simulink library "Renault_Library"  (Simulink 8.2)
settings: Solver=ode3  StartTime=0.0  StopTime=10.0  FixedStep=0.020  RelTol=1e-3
undefined symbols (not defined in the file or in its folder): ABC, AssystemProposal, b, chkval, CoeffA_C, CoeffB_C, CoeffMean_C, ConfTime_C, DiagDelay_C, DividePercent, FailureConfActive_C, FallingEdgeDuration_C, HighLevelThresholdVoltage_C, HighLevelVoltage_C, HighLevelVoltageTunable_C, InitValue_C, InputVoltRangeMax_C, InputVoltRangeMin_C, LowActiveContact_C, LowLevelThresholdVoltage_C, LowLevelVoltage_C, MaxOperatingVoltage_C, MinOperatingVoltage_C, mV2V_conversion, OUT1, OutOfRangeHigh_C, OutOfRangeLow_C, PinSupplySaturationMax, PinSupplySaturationMin, Res1Value_C, Res2Value_C, Res3Value_C, RisingEdgeDuration_C, RSA_CANSignalFailMonitoring, SampleTime_C, service14Table, service19Table, service22Table, service24Table, service28Table, service2ETable, service2FTable, service31Table, service87Table, SizeXStateDID2FTable, SizeXTable14, SizeXTable19, SizeXTable22, SizeXTable24, SizeXTable28, SizeXTable2E, SizeXTable2F, SizeXTable31, SizeXTable87, SizeYStateDID2FTable, SizeYTable14, SizeYTable19, SizeYTable22, SizeYTable24, SizeYTable28 ... (+22)
undefined symbols (not defined in the file): BatteryVoltage_ActivationThreshold_C, FrameStatus_AbsentValue_C, FrameStatus_ConfAbsentValue_C, MemThreshold_C, NAMEOFTHEMODEL_SAMPLE_TIME_CST, NbAbs1_C, NbConfAbs1_C, NbConfPres1_C, NbPres1_C, NOMDUSIGNAL_FaultConfTime_C, NOMDUSIGNAL_FaultRehabTime_C, NOMDUSIGNAL_switchvalue_C, NOMDUSIGNAL_unavailablevalue_C, PeriodFrame1_C, ResetTime_C, WakeUpTime_C
libraries: Renault_Library x112, simulink x75
(part 2 of 32)

== system Renault_Library/Complex blocks/Logic & Bit Operations ==
  note: Logic & Bit Operations
  note: /!\         DO NOT USE THIS RED BLOCKS ANYMORE          /!\
      |
      |
      |
      |
      |
      |
      |
      |
      |
      |
      |
      |
      |
      |
      |
      |
      |
      |
      |
      |
      |
      |
      |
      |
      | .
  RSA_CompareToConstant_V01_04 : Subsystem  Tag=[LIB]  mask(RSA_CompareToConstant){relop===, Const=1, LogicOutDataTypeMode=boolean, ZeroCross=off}
      mask prompts: relop "Operator:"; Const "Constant value:"; LogicOutDataTypeMode "Output data type mode:"; ZeroCross "Enable zero-crossing detection"
      mask help:
      | Output will be one when the signal less than or equal to a constant otherwise it will be zero.
      |
      | The “Operator” parameter can have the following values:
      |       1. "==" — Determine whether the input is equal to Constant.
      |       2. "~=" — Determine whether the input is not equal to Constant.
      |       3. "<"  — Determine whether the input is less than Constant.
      |       4. "<=" — Determine whether the input is less than or equal to Constant.
      |       5. ">"  — Determine whether the input is greater than Constant.
      |       6. ">=" — Determine whether the input is greater than or equal to Constant.
      |
      | Note:
      |       1. Input Datatype of the block must NOT be "Double" and "Single" when "==" or "~="  is used
      |
      |
      | Version : 01.04
      mask init:
      | blkName = gcb;
      | 
      | if strcmp(get_param(gcbh,'LogicOutDataTypeMode'),'boolean')
      | 	setValue = 'boolean';
      | else
      | 	setValue = '''uint8''';
      | end
      | 
      | set_param([blkName,'/Compare'],'relop',relop, ...
      | 	'OutDataTypeStr', setValue, 'ZeroCross', ZeroCross);
      | 
      | str = [relop,' C']; %default
      | 
      | const_str = get_param(blkName, 'Const');
      | 
      | if isempty(strfind(const_str, ' ')) && isempty(strfind(const_str, ','))
      |     % scalar or single expression
      |     if isempty(str2num(const_str))
      |         %expression
      |         str = [relop, ' ', const_str];
      |     elseif length(Const) == 1
      |         str = [relop,' ',num2str(double(Const))];
      |     end
      | end
      settings: AttributesFormatString=%<MaskType> : Ver : 01.04\nOperator: = %<relop>\nConstant value: = %<Const>\nOutput data type mode: = %<LogicOutDataTypeMode>\nEnable zero-crossing detection: = %<ZeroCross>
      mask Help: eval(['open([','''\\F-khepri\shared\DIM-DCMAP-CGMP\66161\Shared_MatLab\Tools_DCMAP\Common_Library_MBDR\Library\Common_Library\Help\RLV_RSA_CompareToConstant_Spec_V1.04.pdf''','])'])
      mask TypeOptions relop: == | ~= | < | <= | >= | >
      mask TypeOptions LogicOutDataTypeMode: uint8 | boolean
      mask Callback ZeroCross: sl('zeroCrossEnable', gcbh);
      mask Display: disp(str)
  RSA_CompareToZero : Subsystem  Tag=[LIB]  mask(RSA_CompareToZero){relop===, LogicOutDataTypeMode=boolean, ZeroCross=on}
      mask prompts: relop "Operator:"; LogicOutDataTypeMode "Output data type mode:"; ZeroCross "Enable zero-crossing detection"
      mask help:
      | RSA_CompareToZero block compares an input signal to zero.
      | Specify how the input is compared to zero with the “Operator” parameter.
      | The “Operator” parameter can have the following values:
      |       1. "==" — Determine whether the input is equal to zero.
      |       2. "~=" — Determine whether the input is not equal to zero.
      |       3. "<"  — Determine whether the input is less than zero.
      |       4. "<=" — Determine whether the input is less than or equal to zero.
      |       5. ">"  — Determine whether the input is greater than zero.
      |       6. ">=" — Determine whether the input is greater than or equal to zero.
      |
      | The output is 0 if the comparison is false, and 1 if it is true.
      |
      | Version : 01.03
      mask init:
      | cons_zero = 0;
      | blkName = gcb;
      | 
      | if strcmp(get_param(gcbh,'LogicOutDataTypeMode'),'boolean')
      | 	setValue = 'boolean';
      | else
      |     setValue = '''uint8''';
      | end
      | 
      | set_param([blkName,'/Compare'],'relop',relop, ...
      | 	'OutDataTypeStr', setValue, 'ZeroCross', ZeroCross);
      settings: AttributesFormatString=%<MaskType> : Ver : 01.03\nOperator: %<relop>\nOutput data type mode: %<LogicOutDataTypeMode>\nEnable zero-crossing detection %<ZeroCross>
      mask Help: eval(['open([','''\\F-khepri\shared\DIM-DCMAP-CGMP\66161\Shared_MatLab\Tools_DCMAP\Common_Library_MBDR\Library\Common_Library\Help\RLV_RSA_CompareToZero_Spec_V1.03.pdf''','])'])
      mask TypeOptions relop: == | ~= | < | <= | >= | >
      mask TypeOptions LogicOutDataTypeMode: uint8 | boolean
      mask Display: disp([relop,' 0'])
  RSA_CompareToZero1 : Subsystem  Tag=[LIB]  mask(RSA_CompareToZero){relop===, LogicOutDataTypeMode=boolean, ZeroCross=on}
      mask prompts: relop "Operator:"; LogicOutDataTypeMode "Output data type mode:"; ZeroCross "Enable zero-crossing detection"
      mask help:
      | RSA_CompareToZero block compares an input signal to zero.
      | Specify how the input is compared to zero with the “Operator” parameter.
      | The “Operator” parameter can have the following values:
      |       1. "==" — Determine whether the input is equal to zero.
      |       2. "~=" — Determine whether the input is not equal to zero.
      |       3. "<"  — Determine whether the input is less than zero.
      |       4. "<=" — Determine whether the input is less than or equal to zero.
      |       5. ">"  — Determine whether the input is greater than zero.
      |       6. ">=" — Determine whether the input is greater than or equal to zero.
      |
      | The output is 0 if the comparison is false, and 1 if it is true.
      |
      | Note:
      |       1. Input Datatype of the block must NOT be "Double" and "Single" when "==" or "~="  is used
      |
      |
      | Version : 01.04
      mask init: same as Renault_Library/Complex blocks/Logic & Bit Operations/RSA_CompareToZero
      settings: AttributesFormatString=%<MaskType> : Ver : 01.04\nOperator: %<relop>\nOutput data type mode: %<LogicOutDataTypeMode>\nEnable zero-crossing detection %<ZeroCross>
      mask Help: eval(['open([','''\\F-khepri\shared\DIM-DCMAP-CGMP\66161\Shared_MatLab\Tools_DCMAP\Common_Library_MBDR\Library\Common_Library\Help\RLV_RSA_CompareToZero_Spec_V1.04.pdf''','])'])
      mask TypeOptions relop: == | ~= | < | <= | >= | >
      mask TypeOptions LogicOutDataTypeMode: uint8 | boolean
      mask Display: disp([relop,' 0'])
  RSA_DetectBooleanChange_v01_0 : Subsystem  Tag=[LIB]  mask(RSA_DetectBooleanChange){chkini=on, vinit=false}
      mask prompts: chkini "Enable first step detection"; vinit "Initial Value :"
      mask help:
      | Detect change in Boolean signal value.
      |
      | If the input does not equal its previous value, then output TRUE, otherwise output FALSE.
      |
      | To inhibit change detection at first simulation step, uncheck "Enable first step detection".
      |
      | Version: 01.0
      mask init:
      | if strcmp(get_param(gcb,'vinit'), 'false')
      |      set_param([gcb '/Delay_Input1'],'InitialCondition','false');
      | else
      |      set_param([gcb '/Delay_Input1'],'InitialCondition','true');
      | end
      settings: AttributesFormatString=|
      | %<MaskType> : Ver : 01.0
      | First step detection = %<chkini>
      | Initial Value : = %<vinit>
      mask Help: eval(['open([','''\\F-khepri\shared\DIM-DCMAP-CGMP\66161\Shared_MatLab\Tools_DCMAP\Common_Library_MBDR\Library\Common_Library\Help\RLV_RSA_DetectBooleanChange_V1_00.pdf''','])'])
      mask Callback chkini:
      | if strcmp(get_param(gcb,'chkini'),'on')
      |         set_param(gcb,'Maskvisibilities',{'on','on'});
      |         set_param(gcb,'attributesFormatString',sprintf('%%<MaskType> : Ver : 01.0\nFirst step detection = %%<chkini>\nInitial Value : = %%<vinit>'));
      | else
      |         set_param(gcb,'Maskvisibilities',{'on','off'});
      |         set_param(gcb,'attributesFormatString',sprintf('%%<MaskType> : Ver : 01.0\nFirst step detection = %%<chkini>'));
      | end
      mask TypeOptions vinit: false | true
      mask Callback vinit:
      | if strcmp(get_param(gcb,'vinit'), 'false')
      |      set_param([gcb '/Delay_Input1'],'InitialCondition','false');
      | else
      |      set_param([gcb '/Delay_Input1'],'InitialCondition','true');
      | end
      mask Display: fprintf(['U ~= U/z' '\nBoolean inputs'])
  RSA_DetectChange : Subsystem  Tag=[LIB]  mask(RSA_DetectChange){vinit=2}
      mask prompts: vinit "Initial condition:"
      mask help:
      | If the input does not equal its previous value, then output TRUE, otherwise output FALSE.  The initial condition determines the initial value of the previous input U/z.
      |
      | Version : 01.02
      settings: AttributesFormatString=%<MaskType> : Ver : 01.02\nInitial condition: %<vinit>
      mask Help: eval(['open([','''\\F-khepri\shared\DIM-DCMAP-CGMP\66161\Shared_MatLab\Tools_DCMAP\Common_Library_MBDR\Library\Common_Library\Help\RLV_RSA_DetectChange_Spec_V1.02.pdf''','])'])
      mask Display: fprintf('U ~= U/z')
  RSA_DetectChange_v01_03 : Subsystem  Tag=[LIB]  mask(RSA_DetectChange){}
      mask help:
      | If the input does not equal its previous value, then output TRUE, otherwise output FALSE.
      |
      | Version : 01.03
      mask init:
      | vinit = 0;
      settings: AttributesFormatString=%<MaskType> : Ver : 01.03
      mask Help: eval(['open([','''\\F-khepri\shared\DIM-DCMAP-CGMP\66161\Shared_MatLab\Tools_DCMAP\Common_Library_MBDR\Library\Common_Library\Help\RLV_RSA_DetectChange_Spec_V1.03.pdf''','])'])
      mask Display: fprintf('U ~= U/z')
  RSA_DetectChange_v01_04 : Subsystem  Tag=[LIB]  mask(RSA_DetectChange){chkini=on, vinit=0}
      mask prompts: chkini "Compare the Input with Initial Value"; vinit "Initial Value :"
      mask help:
      | If the input does not equal its previous value, then output TRUE, otherwise output FALSE.
      |
      | Version : 01.04
      settings: AttributesFormatString=%<MaskType> : Ver : 01.04\nCompare the Input with Initial Value = %<chkini>\nInitial Value : = %<vinit>
      mask Help: eval(['open([','''\\F-khepri\shared\DIM-DCMAP-CGMP\66161\Shared_MatLab\Tools_DCMAP\Common_Library_MBDR\Library\Common_Library\Help\RLV_RSA_DetectChange_Spec_V1.04.pdf''','])'])
      mask Callback chkini:
      | chkval = false;
      | if strcmp(get_param(gcb,'chkini'),'on')
      |     set_param(gcb,'MaskEnables',{'on','on'});
      |     chkval = true;
      | else
      |     set_param(gcb,'MaskEnables',{'on','off'});
      | end
      mask Display: fprintf('U ~= U/z')
  RSA_DetectChange_v01_05 : Subsystem  Tag=[LIB]  mask(RSA_DetectChange){chkini=on, vinit=0, tolerance=Absolute Zero, user_tol=0}
      mask prompts: chkini "Compare the Input with Initial Value"; vinit "Initial Value :"; tolerance "Tolerance for Input"; user_tol "User Defined Tolerance"
      mask help:
      | If the input does not equal its previous value, then output TRUE, otherwise output FALSE.
      | Initial condition of the output is FALSE.
      |
      | NOTE: The check for the initial condition can be inhibited by unchecking the checkbox "Compare the input with Initial Value"
      |
      | Version : 01.05
      mask init:
      | if(strcmp(get_param(gcb,'chkini'),'on'))
      |      set_param([gcb '/From'],'GotoTag','fromRelOpe');
      | else
      |      set_param([gcb '/From'],'GotoTag','vchk_val');
      | end
      | 
      | switch tolerance
      |    case 1
      |        tol=eps('double');
      |    case 2
      |        tol=eps('single');
      |    case 3
      |        tol=0;
      |     case 4
      |        tol = user_tol;
      | end
      settings: AttributesFormatString=|
      | %<MaskType> : Ver : 01.05
      | Compare the Input with Initial Value = %<chkini>
      | Initial Value : = %<vinit>
      | Tolerance : %<tolerance>
      mask Help: eval(['open([','''\\F-khepri\shared\DIM-DCMAP-CGMP\66161\Shared_MatLab\Tools_DCMAP\Common_Library_MBDR\Library\Common_Library\Help\RLV_RSA_DetectChange_Spec_V1.05.pdf''','])'])
      mask Callback chkini:
      | if strcmp(get_param(gcb,'chkini'),'on')
      |     if (strcmp(get_param(gcb,'tolerance'),'User Defined'))
      |         set_param(gcb,'Maskvisibilities',{'on','on','on','on'});
      |         set_param(gcb,'attributesFormatString',sprintf('%%<MaskType> : Ver : 01.05\nCompare the Input with Initial Value = %%<chkini>\nInitial Value : = %%<vinit>\nTolerance : %%<tolerance>\nUser Defined Tol : %%<user_tol>'));
      |     else
      |         set_param(gcb,'Maskvisibilities',{'on','on','on','off'});
      |         set_param(gcb,'attributesFormatString',sprintf('%%<MaskType> : Ver : 01.05\nCompare the Input with Initial Value = %%<chkini>\nInitial Value : = %%<vinit>\nTolerance : %%<tolerance>\n'));
      |     end
      | else
      |     if (strcmp(get_param(gcb,'tolerance'),'User Defined'))
      |         set_param(gcb,'Maskvisibilities',{'on','off','on','on'});
      |         set_param(gcb,'attributesFormatString',sprintf('%%<MaskType> : Ver : 01.05\nCompare the Input with Initial Value = %%<chkini>\nTolerance : %%<tolerance>\nUser Defined Tol : %%<user_tol>'));
      |     else
      |         set_param(gcb,'Maskvisibilities',{'on','off','on','off'});
      |         set_param(gcb,'attributesFormatString',sprintf('%%<MaskType> : Ver : 01.05\nCompare the Input with Initial Value = %%<chkini>\nTolerance : %%<tolerance>\n'));
      |     end
      |     vinit=0;
      | end
      mask TypeOptions tolerance: double tolerance - eps('double') | single tolerance - eps('single') | Absolute Zero | User Defined
      mask Callback tolerance:
      | % if strcmp(get_param(gcb,'chkini'),'on')
      | % set_param(gcb,'AttributesFormatString',sprintf('%%<MaskType> : Ver : 01.05\nCompare the Input with Initial Value = %%<chkini>\nInitial Value : = %%<vinit> \nTolerance : %%<tolerance>'));
      | % else
      | % set_param(gcb,'AttributesFormatString',sprintf('%%<MaskType> : Ver : 01.05\nCompare the Input with Initial Value = %%<chkini> \nTolerance : %%<tolerance>'));
      | % end
      | % switch get_param(gcb,'tolerance')
      | %   case 'double tolerance - eps(''double'')'
      | %       set_param(gcb,'MaskVisibilities',{'on',get_param(gcb,'chkini'), 'on','off'});
      | %   case 'single tolerance - eps(''single'')'
      | %       set_param(gcb,'MaskVisibilities',{'on',get_param(gcb,'chkini'), 'on','off'});
      | %   case 'Absolute Zero'
      | %       set_param(gcb,'MaskVisibilities',{'on',get_param(gcb,'chkini'), 'on','off'});
      | %    case'User Defined'
      | %        if strcmp(get_param(gcb,'chkini'),'on')
      | %        set_param(gcb,'AttributesFormatString',sprintf('%%<MaskType> : Ver : 01.05\nCompare the Input with Initial Value = %%<chkini> \nInitial Value : = %%<vinit> \nTolerance : %%<tolerance> \nUser Defined Tol : %%<user_tol>'));
      | %        else
      | %        set_param(gcb,'AttributesFormatString',sprintf('%%<MaskType> : Ver : 01.05\nCompare the Input with Initial Value = %%<chkini> \nTolerance : %%<tolerance> \nUser Defined Tol : %%<user_tol>'));
      | %        end
      | %        set_param(gcb,'MaskVisibilities',{'on',get_param(gcb,'chkini'), 'on','on'});
      | %
      | % end
      | 
      | 
      | if(strcmp(get_param(gcb,'chkini'),'on'))
      |     if (strcmp(get_param(gcb,'tolerance'),'User Defined'))
      |         set_param(gcb,'Maskvisibilities',{'on','on','on','on'});
      |         set_param(gcb,'attributesFormatString',sprintf('%%<MaskType> : Ver : 01.05\nCompare the Input with Initial Value = %%<chkini>\nInitial Value : = %%<vinit>\nTolerance : %%<tolerance>\nUser Defined Tol : %%<user_tol>'));
      |     else
      |         set_param(gcb,'Maskvisibilities',{'on','on','on','off'});
      |         set_param(gcb,'attributesFormatString',sprintf('%%<MaskType> : Ver : 01.05\nCompare the Input with Initial Value = %%<chkini>\nInitial Value : = %%<vinit>\nTolerance : %%<tolerance>\n'));
      |     end
      | else
      |     if (strcmp(get_param(gcb,'tolerance'),'User Defined'))
      |         set_param(gcb,'Maskvisibilities',{'on','off','on','on'});
      |         set_param(gcb,'attributesFormatString',sprintf('%%<MaskType> : Ver : 01.05\nCompare the Input with Initial Value = %%<chkini>\nTolerance : %%<tolerance>\nUser Defined Tol : %%<user_tol>'));
      |     else
      |         set_param(gcb,'Maskvisibilities',{'on','off','on','off'});
      |         set_param(gcb,'attributesFormatString',sprintf('%%<MaskType> : Ver : 01.05\nCompare the Input with Initial Value = %%<chkini>\nTolerance : %%<tolerance>\n'));
      |     end
      | end
      mask Display: disp('U ~= U/z');
  RSA_DetectDecrease : Subsystem  Tag=[LIB]  mask(RSA_DetectDecrease){vinit=2}
      mask prompts: vinit "Initial condition:"
      mask help:
      | If the input is strictly less than its previous value, then output TRUE, otherwise output FALSE.  The initial condition determines the initial value of the previous input U/z.
      |
      | Version : 01.02
      settings: AttributesFormatString=%<MaskType> : Ver : 01.02\nInitial condition: %<vinit>
      mask Help: eval(['open([','''\\F-khepri\shared\DIM-DCMAP-CGMP\66161\Shared_MatLab\Tools_DCMAP\Common_Library_MBDR\Library\Common_Library\Help\RLV_RSA_DetectDecrease_Spec_V1.02.pdf''','])'])
      mask Display: fprintf('U < U/z')
  RSA_DetectDecrease_v01_03 : Subsystem  Tag=[LIB]  mask(RSA_DetectDecrease){}
      mask help:
      | If the input is strictly less than its previous value, then output TRUE, otherwise output FALSE.  Initial condition of the output is false.
      |
      | Version : 01.03
      mask init:
      | vinit = 0;
      settings: AttributesFormatString=%<MaskType> : Ver : 01.03
      mask Help: eval(['open([','''\\F-khepri\shared\DIM-DCMAP-CGMP\66161\Shared_MatLab\Tools_DCMAP\Common_Library_MBDR\Library\Common_Library\Help\RLV_RSA_DetectDecrease_Spec_V1.03.pdf''','])'])
      mask Display: fprintf('U < U/z')
  RSA_DetectDecrease_v01_04 : Subsystem  Tag=[LIB]  mask(RSA_DetectDecrease){chkini=on, vinit=0}
      mask prompts: chkini "Compare the Input with Initial Value"; vinit "Initial Value :"
      mask help:
      | If the input is strictly less than its previous value, then output TRUE, otherwise output FALSE.  Initial condition of the output is false.
      |
      | WARNING: THIS BLOCK HAS BECOME OBSOLETE. PLEASE USE RSA_DetectIncDec_V01_00 WITH DETECT CHANGE PARAMETER AS DETECT DECREASE!!!
      |
      | Version : 01.04
      settings: AttributesFormatString=%<MaskType> : Ver : 01.04\nCompare the Input with Initial Value = %<chkini>\nInitial Value : = %<vinit>
      mask Help: eval(['open([','''\\F-khepri\shared\DIM-DCMAP-CGMP\66161\Shared_MatLab\Tools_DCMAP\Common_Library_MBDR\Library\Common_Library\Help\RLV_RSA_DetectDecrease_Spec_V1.04.pdf''','])'])
      mask Callback chkini:
      | chkval = false;
      | if(strcmp(get_param(gcb,'chkini'),'on'))
      |     set_param(gcb,'MaskEnables',{'on','on'});
      |     chkval = true;
      | else
      |     set_param(gcb,'MaskEnables',{'on','off'});
      | end
      mask Display: fprintf('U < U/z')
  RSA_DetectIncDec_V01_00 : Subsystem  Tag=[LIB]  mask(RSA_DetectIncDec){Detect_chg=Detect Decrease, chkini=off, vinit=0}
      mask prompts: Detect_chg "Detect Change Input"; chkini "Compare the Input with Initial Value"; vinit "Initial Value :"
      mask help:
      | Detect Decrease:
      | If the input is strictly lesser than its previous value, then output TRUE, otherwise output FALSE. Initial condition of the output is false.
      | Detect Increase:
      | If the input is strictly greater than its previous value, then output TRUE, otherwise output FALSE. Initial condition of the output is false.
      |
      | NOTE:
      | The check for the initial condidtion can be inhibited by unchecking the checkbox
      | "Compare the Input with Initial Value".
      |
      | Version : 01.00
      mask init:
      | switch Detect_chg
      |     case 1
      |         set_param([gcb '/FixPt_Relational_Operator'],'Operator','>');
      |     case 2
      |         set_param([gcb '/FixPt_Relational_Operator'],'Operator','<');
      | end
      | 
      | if(strcmp(get_param(gcb,'chkini'),'on'))
      |      set_param([gcb '/From'],'GotoTag','fromRelOpe');
      | else
      |      set_param([gcb '/From'],'GotoTag','vchk_val');
      | end
      settings: AttributesFormatString=|
      | %<MaskType> : Ver : 01.00
      | Detect Type : %<Detect_chg>
      | Compare the Input with Initial Value = %<chkini>
      mask Help: eval(['open([','''\\F-khepri\shared\DIM-DCMAP-CGMP\66161\Shared_MatLab\Tools_DCMAP\Common_Library_MBDR\Library\Common_Library\Help\RLV_RSA_DetectIncDec_Spec_V1.00.pdf''','])'])
      mask TypeOptions Detect_chg: Detect Increase | Detect Decrease
      mask Callback chkini:
      | chkval = false;
      | set_param(gcb,'attributesFormatString',sprintf('%%<MaskType> : Ver : 01.00\nDetect Type : %%<Detect_chg>\nCompare the Input with Initial Value = %%<chkini>\nInitial Value : = %%<vinit>'));
      | 
      | if(strcmp(get_param(gcb,'chkini'),'on'))
      |     set_param(gcb,'Maskvisibilities',{'on','on','on'});
      |     chkval = true;
      | else
      |     set_param(gcb,'Maskvisibilities',{'on','on','off'});
      |     vinit=0;
      |     set_param(gcb,'attributesFormatString',sprintf('%%<MaskType> : Ver : 01.00\nDetect Type : %%<Detect_chg>\nCompare the Input with Initial Value = %%<chkini>'));
      | end
      mask Display:
      | if Detect_chg==2
      |     fprintf('U < U/z')
      | else
      |     fprintf('U > U/z')
      | end
  RSA_DetectIncrease : Subsystem  Tag=[LIB]  mask(RSA_DetectIncrease){vinit=2}
      mask prompts: vinit "Initial condition:"
      mask help:
      | If the input is strictly greater than its previous value, then output TRUE, otherwise output FALSE.  The initial condition determines the initial value of the previous input U/z.
      |
      | Version : 01.02
      settings: AttributesFormatString=%<MaskType> : Ver : 01.02\nInitial condition: %<vinit>
      mask Help: eval(['open([','''\\F-khepri\shared\DIM-DCMAP-CGMP\66161\Shared_MatLab\Tools_DCMAP\Common_Library_MBDR\Library\Common_Library\Help\RLV_RSA_DetectIncrease_Spec_V1.02.pdf''','])'])
      mask Display: fprintf('U > U/z')
  RSA_DetectIncrease_v01_03 : Subsystem  Tag=[LIB]  mask(RSA_DetectIncrease){}
      mask help:
      | If the input is strictly greater than its previous value, then output TRUE, otherwise output FALSE. Initial condition of the output is false.
      |
      | Version : 01.03
      mask init:
      | vinit = 0;
      settings: AttributesFormatString=%<MaskType> : Ver : 01.03
      mask Help: eval(['open([','''\\F-khepri\shared\DIM-DCMAP-CGMP\66161\Shared_MatLab\Tools_DCMAP\Common_Library_MBDR\Library\Common_Library\Help\RLV_RSA_DetectIncrease_Spec_V1.03.pdf''','])'])
      mask Display: fprintf('U > U/z')
  RSA_DetectIncrease_v01_04 : Subsystem  Tag=[LIB]  mask(RSA_DetectIncrease){chkini=on, vinit=0}
      mask prompts: chkini "Compare the Input with Initial Value"; vinit "Initial Value :"
      mask help:
      | If the input is strictly greater than its previous value, then output TRUE, otherwise output FALSE. Initial condition of the output is false.
      |
      | WARNING: THIS BLOCK HAS BECOME OBSOLETE. PLEASE USE RSA_DetectIncDec_V01_00 WITH DETECT CHANGE PARAMETER AS DETECT INCREASE!!!
      |
      | Version : 01.04
      settings: AttributesFormatString=%<MaskType> : Ver : 01.04\nCompare the Input with Initial Value = %<chkini>\nInitial Value : = %<vinit>
      mask Help: eval(['open([','''\\F-khepri\shared\DIM-DCMAP-CGMP\66161\Shared_MatLab\Tools_DCMAP\Common_Library_MBDR\Library\Common_Library\Help\RLV_RSA_DetectIncrease_Spec_V1.04.pdf''','])'])
      mask Callback chkini:
      | chkval = false;
      | if strcmp(get_param(gcb,'chkini'),'on')
      |     set_param(gcb,'MaskEnables',{'on','on'});
      |     chkval = true;
      | else
      |     set_param(gcb,'MaskEnables',{'on','off'});
      | end
      mask Display: fprintf('U > U/z')
  RSA_EdgeDetect : Subsystem  Tag=[LIB]  mask(RSA_EdgeDetect){Edge_type=Rising, St=-1}
      mask prompts: Edge_type "Hit crossing direction"; St "Sample time (-1 for inherited)"
      mask help:
      | This block can be used to :
      | 	* Detect change
      | 	* Detect increase
      | 	* Detect decrease
      |
      | The initial condition is given by the signal IC.
      | The output is a signal of data type 'boolean'.
      |
      | Version : 01.01
      mask init:
      | a=0.2;
      | b=0.4;
      | c=0.6;
      | d=0.6;
      | e=0.9;
      | 
      | if Edge_type==1	%Rising
      | a=0.2;
      | b=0.4;
      | c=0.6;
      | d=0.6;
      | e=0.9;
      | f=0.6;
      | g=0.9;
      | elseif Edge_type==2	%Falling
      | a=0.2;
      | b=0.4;
      | c=0.6;
      | d=0.9;
      | e=0.6;
      | f=0.9;
      | g=0.6;
      | else	%Either
      | a=0.2;
      | b=0.4;
      | c=0.6;
      | d=0.9;
      | e=0.6;
      | f=0.6;
      | g=0.9;
      | end
      settings: AttributesFormatString=%<MaskType> : Ver : 01.01\n<%<Edge_type>>
      mask Help: eval(['open([','''\\F-khepri\shared\DIM-DCMAP-CGMP\66161\Shared_MatLab\Tools_DCMAP\Common_Library_MBDR\Library\Common_Library\Help\RLV_RSA_EdgeDetect_Spec_V1.01.pdf''','])'])
      mask TypeOptions Edge_type: Rising | Falling | Either
      mask Display:
      | color('green');
      | plot([a b b c],[f f g g]);
      | color('red');
      | plot([a b b c],[d d e e]);
      | color('blue');
      | plot([a b b b c],[0.1 0.1 0.4 0.1 0.1])
  RSA_EdgeDetect_V01_02 : Subsystem  Tag=[LIB]  mask(RSA_EdgeDetect){Edge_type=Falling}
      mask prompts: Edge_type "Hit crossing direction"
      mask help:
      | This block can be used to :
      | 	* Detect change
      | 	* Detect increase
      | 	* Detect decrease
      |
      | The initial condition is given by the signal IC.
      | The output is a signal of data type 'boolean'.
      |
      | Note : Both inputs should be "boolean" DataType.
      | Version : 01.02
      mask init:
      | a=0.2;
      | b=0.4;
      | c=0.6;
      | d=0.6;
      | e=0.9;
      | 
      | if Edge_type==1	%Rising
      | set_param([gcb '/VarSelect'], 'GotoTag', 'RisingEdge');
      | a=0.2;
      | b=0.4;
      | c=0.6;
      | d=0.6;
      | e=0.9;
      | f=0.6;
      | g=0.9;
      | elseif Edge_type==2	%Falling
      | set_param([gcb '/VarSelect'], 'GotoTag', 'FallingEdge');
      | a=0.2;
      | b=0.4;
      | c=0.6;
      | d=0.9;
      | e=0.6;
      | f=0.9;
      | g=0.6;
      | else	%Either
      | set_param([gcb '/VarSelect'], 'GotoTag', 'Either');
      | a=0.2;
      | b=0.4;
      | c=0.6;
      | d=0.9;
      | e=0.6;
      | f=0.6;
      | g=0.9;
      | end
      settings: AttributesFormatString=|
      | %<MaskType> : Ver : 01.02
      | Edge Type : <%<Edge_type>>
      mask Help: eval(['open([','''\\F-khepri\shared\DIM-DCMAP-CGMP\66161\Shared_MatLab\Tools_DCMAP\Common_Library_MBDR\Library\Common_Library\Help\RLV_RSA_EdgeDetect_Spec_V1.02.pdf''','])'])
      mask TypeOptions Edge_type: Rising | Falling | Either
      mask Display:
      | color('green');
      | plot([a b b c],[f f g g]);
      | color('red');
      | plot([a b b c],[d d e e]);
      | color('blue');
      | plot([a b b b c],[0.1 0.1 0.4 0.1 0.1])

== system Renault_Library/Complex blocks/Lookup Tables ==
  note: Lookup Tables
  note: /!\         DO NOT USE THIS RED BLOCKS ANYMORE          /!\
      |
      |
      |
      |
      |
      |
      |
      |
      |
      |
      |
      |
      |
      |
      |
      |
      |
      |
      |
      |
      |
      |
      |
      |
      |
      |
      |
      |
      |
      |
      |
      |
      |
      |
      |
      |
      |
      |
      |
      |
      |
      |
      |
      |
      |
      |
      |
      |
      |
      |
      |
      |
      |
      |
      |
      |
      |
      |
      |
      |
      |
      |
      |
      |
      |
      |
      |
      |
      |
      |
      |
      |
      |
      |
      |
      |
      |
      |
      |
      |
      |
      |
      |
      | .
  RSA_Cosine_v01_03 : Subsystem  Tag=[LIB]  mask(RSA_Cosine){NumDataPoints=32, OutputWordLength=8}
      mask prompts: NumDataPoints "Number of data points for Lookup table:"; OutputWordLength "Output Word Length:"
      mask help:
      | The block implements a Cosine wave using lookup table method that exploits quarter wave symmetry.
      |
      | Note:
      | Matlab variable pi and matlab function Power,factorial is used in mask initialization. Accuracy is needed more to represent those functions values.
      |
      | Version : 01.03
      mask init:
      | INIVAL = 0;
      | NVAL = -1;
      | 
      | k=linspace(0,0.25,NumDataPoints);
      | for i = 1:NumDataPoints
      |     l = 0;
      |     b(i) = 0;
      |     act_poin=double(i);
      |     %k(i) = 2*pi* (act_poin-1)*0.25;   
      |     for j = 1:2:63
      |         l = l+1;
      |         if(rem(l,2))
      |             b(i) = b(i) + (power(k(i)*2*pi,j)/factorial(j));
      |         else
      |             b(i) = b(i) - (power(k(i)*2*pi,j)/factorial(j));
      |         end
      |     end
      | end
      | OutputFracLength = OutputWordLength-2;
      settings: AttributesFormatString=%<MaskType> : Ver : 01.03\nNumber of data points for Lookup table: = %<NumDataPoints>\nOutput Word Length: = %<OutputWordLength>
      mask Help: eval(['open([','''\\F-khepri\shared\DIM-DCMAP-CGMP\66161\Shared_MatLab\Tools_DCMAP\Common_Library_MBDR\Library\Common_Library\Help\RLV_RSA_Cosine_Spec_V1.03.pdf''','])'])
  RSA_Cosine_v01_04 : Subsystem  Tag=[LIB]  mask(RSA_Cosine){NumDataPoints=32, OutputWordLength=8}
      mask prompts: NumDataPoints "Number of data points for Lookup table:"; OutputWordLength "Output Word Length:"
      mask help:
      | The block implements a Cosine wave in fixed point using lookup table method that exploits quarter wave symmetry.
      |
      | Note:
      | Matlab variable pi and matlab function Power,factorial is used in mask initialization. Accuracy is needed more to represent those functions values.
      |
      | Version : 01.04
      mask init:
      | INIVAL = 0;
      | CONS_P25=0.25;
      | CONS_P5=0.5;
      | CONS_P75=0.75;
      | CONS_M1 = -1;
      | 
      | k=linspace(0,0.25,NumDataPoints);
      | for i = 1:NumDataPoints
      |     l = 0;
      |     b(i) = 0;
      |     act_poin=double(i);
      |     %k(i) = 2*pi* (act_poin-1)*0.25;   
      |     for j = 1:2:63
      |         l = l+1;
      |         if(rem(l,2))
      |             b(i) = b(i) + (power(k(i)*2*pi,j)/factorial(j));
      |         else
      |             b(i) = b(i) - (power(k(i)*2*pi,j)/factorial(j));
      |         end
      |     end
      | end
      | OutputFracLength = OutputWordLength-2;
      settings: AttributesFormatString=%<MaskType> : Ver : 01.04\nNumber of data points for Lookup table: = %<NumDataPoints>\nOutput Word Length: = %<OutputWordLength>
      mask Help: eval(['open([','''\\F-khepri\shared\DIM-DCMAP-CGMP\66161\Shared_MatLab\Tools_DCMAP\Common_Library_MBDR\Library\Common_Library\Help\RLV_RSA_Cosine_Spec_V1.04.pdf''','])'])
  RSA_LookupTableDynamic_v02_00 : Subsystem  Tag=[LIB]  mask(RSA_LookupTableDynamic){datt=fixdt(0,32,0.001,0)}
      mask prompts: datt "Data type for division operation :"
      mask help:
      | The Lookup Table Dynamic block computes an approximation of a function y = f(x) using xdat and ydat vectors. The lookup method can use interpolation or the original values of the input.
      | Using the Lookup Table Dynamic block, the table data can be changed without stopping the simulation.
      |
      | The Values for xdat should be strictly monotonical increasing order.
      |
      | The rounding method 'zero' is used for all arithmetic calculations.
      |
      | Version : 02.00
      mask init:
      | cons1 = 1;
      | divchk = 0;
      settings: AttributesFormatString=%<MaskType> : Ver : 02.00\nData type for division operation : = %<datt>
      mask Help: eval(['open([','''\\F-khepri\shared\DIM-DCMAP-CGMP\66161\Shared_MatLab\Tools_DCMAP\Common_Library_MBDR\Library\Common_Library\Help\RLV_RSA_LookupTableDynamic_Spec_V2.00.pdf''','])'])
  RSA_LookupTableDynamic_v02_01 : Subsystem  Tag=[LIB]  mask(RSA_LookupTableDynamic){datt=double, tolerance=Absolute Zero, user_tol=0}
      mask prompts: datt "Data type for division operation :"; tolerance "Tolerance for Division"; user_tol "User Tolerance"
      mask help:
      | The Lookup Table Dynamic block computes an approximation of a function y = f(x) using xdat and ydat vectors. The lookup method can use interpolation or the original values of the input.
      | Using the Lookup Table Dynamic block, the table data can be changed without stopping the simulation.
      |
      | The Values for xdat should be strictly monotonical increasing order.
      |
      | The rounding method 'zero' is used for all arithmetic calculations.
      |
      | Version : 02.01
      mask init:
      | cons1 = 1;
      | cons0 = 0;
      | divchk = 0;
      | switch tolerance
      |    case 1
      |        divchk=eps('double');
      |    case 2
      |        divchk=eps('single');
      |    case 3
      |        divchk=0;
      |    case 4
      |        divchk = user_tol;
      | end
      settings: AttributesFormatString=|
      | %<MaskType> : Ver : 02.01
      | Datatype For Division : %<datt>
      | Tolerance : %<tolerance>
      mask Help: eval(['open([','''\\F-khepri\shared\DIM-DCMAP-CGMP\66161\Shared_MatLab\Tools_DCMAP\Common_Library_MBDR\Library\Common_Library\Help\RLV_RSA_LookupTableDynamic_Spec_V2.01.pdf''','])'])
      mask TypeOptions tolerance: eps Double | eps Single | Absolute Zero | User Defined
      mask Callback tolerance:
      | set_param(gcb,'AttributesFormatString',sprintf('%%<MaskType> : Ver : 02.01\nDatatype For Division : %%<datt>\nTolerance : %%<tolerance>'));
      | switch get_param(gcb,'tolerance')
      |   case 'eps Double'
      |       set_param(gcb,'MaskVisibilities',{'on','on', 'off'});
      |   case 'eps Single'
      |       set_param(gcb,'MaskVisibilities',{'on','on', 'off'});
      |   case 'Absolute Zero'
      |       set_param(gcb,'MaskVisibilities',{'on','on', 'off'});
      |    case'User Defined'
      |        set_param(gcb,'AttributesFormatString',sprintf('%%<MaskType> : Ver : 02.01\nDatatype For Division : %%<datt>\nTolerance : %%<tolerance>\nUser defined Tol : %%<user_tol>'));
      |        set_param(gcb,'MaskVisibilities',{'on','on', 'on'});
      | end
  RSA_Sine_v01_03 : Subsystem  Tag=[LIB]  mask(RSA_Sine){NumDataPoints=32, OutputWordLength=16}
      mask prompts: NumDataPoints "Number of data points for lookup table:"; OutputWordLength "Output word length:"
      mask help:
      | The block implements a sine wave using lookup table method that exploits quarter wave symmetry.
      |
      | Note:
      | Matlab variable pi and matlab function Power,factorial is used in mask initialization. Accuracy is needed more to represent those functions values.
      |
      | Version : 01.03
      mask init:
      | k=linspace(0,0.25,NumDataPoints);
      | for i = 1:NumDataPoints
      |     l = 0;
      |     b(i) = 0;
      |     act_poin=double(i);
      |     %k(i) = 2*pi* (act_poin-1)*0.25;   
      |     for j = 1:2:63
      |         l = l+1;
      |         if(rem(l,2))
      |             b(i) = b(i) + (power(k(i)*2*pi,j)/factorial(j));
      |         else
      |             b(i) = b(i) - (power(k(i)*2*pi,j)/factorial(j));
      |         end
      |     end
      | end
      | OutputFracLength = OutputWordLength-2;
      settings: AttributesFormatString=%<MaskType> : Ver : 01.03\nNumber of data points for lookup table: = %<NumDataPoints>\nOutput word length: = %<OutputWordLength>
      mask Help: eval(['open([','''\\F-khepri\shared\DIM-DCMAP-CGMP\66161\Shared_MatLab\Tools_DCMAP\Common_Library_MBDR\Library\Common_Library\Help\RLV_RSA_Sine_Spec_V1.03.pdf''','])'])
  RSA_Sine_v01_04 : Subsystem  Tag=[LIB]  mask(RSA_Sine){NumDataPoints=32, OutputWordLength=16}
      mask prompts: NumDataPoints "Number of data points for lookup table:"; OutputWordLength "Output word length:"
      mask help:
      | The block implements a sine wave using lookup table method that exploits quarter wave symmetry.
      |
      | Note:
      | Matlab variable pi and matlab function Power,factorial is used in mask initialization. Accuracy is needed more to represent those functions values.
      |
      | Version : 01.04
      mask init:
      | CONS_P25=0.25;
      | CONS_P5=0.5;
      | CONS_M1=-1;
      | k=linspace(0,0.25,NumDataPoints);
      | for i = 1:NumDataPoints
      |     l = 0;
      |     b(i) = 0;
      |     act_poin=double(i);
      |     %k(i) = 2*pi* (act_poin-1)*0.25;   
      |     for j = 1:2:63
      |         l = l+1;
      |         if(rem(l,2))
      |             b(i) = b(i) + (power(k(i)*2*pi,j)/factorial(j));
      |         else
      |             b(i) = b(i) - (power(k(i)*2*pi,j)/factorial(j));
      |         end
      |     end
      | end
      | OutputFracLength = OutputWordLength-2;
      settings: AttributesFormatString=%<MaskType> : Ver : 01.04\nNumber of data points for lookup table: = %<NumDataPoints>\nOutput word length: = %<OutputWordLength>
      mask Help: eval(['open([','''\\F-khepri\shared\DIM-DCMAP-CGMP\66161\Shared_MatLab\Tools_DCMAP\Common_Library_MBDR\Library\Common_Library\Help\RLV_RSA_Sine_Spec_V1.04.pdf''','])'])

== system Renault_Library/Complex blocks/Math Operations ==
  note: Math Operations
  note: /!\         DO NOT USE THIS RED BLOCKS ANYMORE          /!\
      |
      |
      |
      |
      |
      |
      |
      |
      |
      |
      |
      |
      |
      |
      |
      |
      |
      |
      |
      |
      |
      |
      |
      |
      | .
  RSA_ADivB : Subsystem  Tag=[LIB]  mask(RSA_ADivB){}
      mask help:
      | This block gives output "AdivB" by dividing two input "A" and "B".
      | (Note:If "B"=0, it will be replaced by "eps").
      |
      | Note:
      | Since matlab variable 'eps' is used inside this block,
      | so accuracy is needed for the input values.
      |
      | Version : 01.02
      settings: AttributesFormatString=%<MaskType> : Ver : 01.02
      mask Help:
      | This block gives output "AdivB" by dividing two input "A" and "B".
      | (Note:If "B"=0, it will be replaced by "eps").
      | 
      | Note:
      | Since matlab variable 'eps' is used inside this block,
      | so accuracy is needed for the input values.
      | 
      | Version : 01.02
  RSA_DivisionByZero : Subsystem  Tag=[LIB]  mask(RSA_DivisionByZero){}
      mask help:
      | This block outputs the value of the division operation "num / den ". If the "den" is 0,the output will be the value at the port "ans_of_0div"
      |
      | Version : 01.03
      mask init:
      | DIVBY0 = 0;
      settings: AttributesFormatString=%<MaskType> : Ver : 01.03
      mask Help: eval(['open([','''\\F-khepri\shared\DIM-DCMAP-CGMP\66161\Shared_MatLab\Tools_DCMAP\Common_Library_MBDR\Library\Common_Library\Help\RLV_RSA_DivisionByZero_Spec_V1.03.pdf''','])'])
      mask Display:
      | port_label('input',1,'den')
      | port_label('input',2,'num')
      | port_label('output',1,'result')
      | port_label('input',3,'ans_of_0div')
      | disp('Division \n no division by "0"')
  RSA_DivisionByZero_v01_04 : Subsystem  Tag=[LIB]  mask(RSA_DivisionByZero){}
      mask help:
      | This block outputs the value of the division operation "num / den ". If the "den" is 0,the output will be the value at the port "ans_of_0div"
      |
      | Version : 01.04
      mask init:
      | DIVBY0 = 0;
      settings: AttributesFormatString=%<MaskType> : Ver : 01.04
      mask Help: eval(['open([','''\\F-khepri\shared\DIM-DCMAP-CGMP\66161\Shared_MatLab\Tools_DCMAP\Common_Library_MBDR\Library\Common_Library\Help\RLV_RSA_DivisionByZero_Spec_V1.04.pdf''','])'])
      mask Display:
      | port_label('input',1,'den')
      | port_label('input',2,'num')
      | port_label('output',1,'result')
      | port_label('input',3,'ans_of_0div')
      | disp('Division \n no division by "0"')
  RSA_DivisionByZero_v01_05 : Subsystem  Tag=[LIB]  mask(RSA_DivisionByZero){Den_Tol=eps}
      mask prompts: Den_Tol "Denominator Tolerance"
      mask help:
      | This block outputs the value of the division operation "num / den ". If the "den" is less than "Denominator Tolerance",the output will be the value at the port "ans_of_0div".
      |
      | Note : For TargetLink Code generation, "protect against division by zero in production code" property should be disabled for the divide block present inside this Library.
      |
      | Version : 01.05
      settings: AttributesFormatString=%<MaskType> : Ver : 01.05\nDenominator tolerance : %<Den_Tol>
      mask Help: eval(['open([','''\\F-khepri\shared\DIM-DCMAP-CGMP\66161\Shared_MatLab\Tools_DCMAP\Common_Library_MBDR\Library\Common_Library\Help\RLV_RSA_DivisionByZero_Spec_V1.05.pdf''','])'])
      mask Display:
      | port_label('input',1,'den')
      | port_label('input',2,'num')
      | port_label('output',1,'result')
      | port_label('input',3,'ans_of_0div')
      | disp('Division \n no division by "0"')
  RSA_Modulo_V01_00 : Subsystem  Tag=[LIB]  mask(RSA_Modulo){InternalDataType=uint16, Den=Absolute Zero Tol, User_Value=0, chkdt=off}
      mask prompts: InternalDataType "Data Type for output of Division"; Den "Tolerance For Floating Point Comparison To Zero"; User_Value "User Defined Tolerance Value"; chkdt "Input of Unsigned Datatype"
      mask help:
      | Description
      | M = mod(X,Y) returns the modulus after division of X by Y.
      |
      | If Y does not equal 0, M = mod(X,Y) returns X - n.*Y, where n = floor(X./Y)
      | Inputs X and Y must have the same dimensions unless one is a scalar double.
      | If one input has an integer data type, then the other input must be of the same integer data type or be a scalar double.
      |
      | <Data Type For Internal Calculations> parameter defines possible data types for the operation x./y
      | Tune this parameter as per max and min possible values of x and y;
      |  - If uint8 is selected, the block calculates correct results up to the value of the ratio x./y is <= intmax('uint8');
      |  - If uint16 is selected, the block calculates correct results up to the value of the ratio x./y is <= intmax('uint16');
      |  - If uint32 is selected, the block calculates correct results up to the value of the ratio x./y is <= intmax('uint32');
      |
      | <Tolerance For Floating Point Comparison To Zero> : Tune this parameter as follows :
      |   - Select <Double Tol> if block’s inputs are of Double data type (eps('double'))
      |   - Select <Single Tol > if block’s inputs are of Single data type (eps('single'))
      |   - Select <Absolute Zero Tol> if block’s inputs are of Integer of fixdt datatype (0)
      |   - Select <User Defined Tol> to define custom tolerance value.
      | If the selected value is <User Defined Tol> the parameters <User Division Tolerance Value> and <User Value DataType> are enabled. The data type must be the same as block’s inputs data type, select <Inherit : ….> to inherit this data type from block’s inputs if defined.
      | An error occurs if tolerance selection is not performed as specified above.
      | An error occurs if inputs are not of the same data type.
      |
      | Also ,
      | 1)The two inputs x and y must have the same data type.
      | 2)The result of Abs(X)/Abs(Y) should not exceed the Maximum value of the data type selected in "<Data Type For Internal Calculations>"
      | 3)For inputs with signed data type, Minimum value of the signed data type should not be passed as input.
      | 4)For RTW code generation select "Remove code that protects against division arithmetic exceptions" option in Configuration Parameters settings.
      |
      | The following are true by convention:
      | - mod(X,0) is X
      | - mod(X,X) is 0
      | - mod(X,Y) for X~=Y and Y~=0 has the same sign as Y
      |
      | Version : 01.00
      mask init:
      | cmpTol = 0;
      | cmpZero=0;
      | cste0  = 0;
      | constOne = 1;
      | 
      | if(strcmp(get_param(gcb,'chkdt'),'on'))
      |      set_param([gcb '/From19'],'GotoTag','constone');
      | else
      |      set_param([gcb '/From19'],'GotoTag','Mulconst');
      | end
      | 
      | switch Den
      |    case 'Double Tol'
      |        cmpTol=eps('double');
      |    case 'Single Tol'
      |        cmpTol=eps('single');
      |    case 'Absolute Zero Tol'
      |        cmpTol=0;
      |     case'User Defined Tol'
      |        cmpTol = User_Value;
      | end
      settings: AttributesFormatString=|
      | %<MaskType> : V01.00
      | DataType For Division : %<InternalDataType>
      | Tolerance : %<Den>
      |  UnsignedDataType : %<chkdt>
      mask Help: eval(['open([','''\\F-khepri\shared\DIM-DCMAP-CGMP\66161\Shared_MatLab\Tools_DCMAP\Common_Library_MBDR\Library\Common_Library\Help\RLV_RSA_Modulo_Spec_V1.00.pdf''','])'])
      mask TypeOptions Den: Double Tol | Single Tol | Absolute Zero Tol | User Defined Tol
      mask Callback Den:
      | %denVal=get_param(gcb,'Den');
      | set_param(gcb,'attributesformatstring',sprintf('%%<MaskType> : V01.00\nDataType For Division : %%<InternalDataType>\nTolerance : %%<Den> \n UnsignedDataType : %%<chkdt>'));
      | switch get_param(gcb,'Den')
      |   case 'Double Tol'
      |       set_param(gcb,'MaskVisibilities',{'on','on','off','on'});
      |   case 'Single Tol'
      |       set_param(gcb,'MaskVisibilities',{'on','on','off','on'});
      |   case 'Absolute Zero Tol'
      |       set_param(gcb,'MaskVisibilities',{'on','on','off','on'});
      |    case'User Defined Tol'
      |        set_param(gcb,'MaskVisibilities',{'on','on', 'on','on'});
      |        set_param(gcb,'attributesformatstring',sprintf('%%<MaskType> : V01.00\nDataType For Division : %%<InternalDataType>\nTolerance : %%<Den> & Value : %%<User_Value> \n UnsignedDataType : %%<chkdt>'));
      | end
      mask Display: disp('M=mod(X,Y)');
